simulink model "BaseEngineController_A02"  (Simulink 7.7)
settings: Solver=FixedStepDiscrete  StartTime=0.0  StopTime=10.0  RelTol=1e-3
PreLoadFcn:
  | try, eval([bdroot '_setup']); catch, eval('model_setup', ''); end
InitFcn:
  | try, eval([bdroot '_setup']); catch, eval('model_setup', ''); end
undefined symbols (not defined in the file or in its folder): ECU555, High, motohawk_build_version, motohawk_get_faults, motohawk_trunc_name, motohawk_vardec_config_group, motohawk_vardec_path, True
libraries: MotoHawk_lib x1145, AutoDoc_lib x786, motohawk_control_primitives_lib x114, motohawk_engine_lib x19, motohawk_annotations_lib x12, simulink x11, simulink_extras x1
(part 12 of 23)

== system BaseEngineController_A02/Foreground/Control/Controller/Run/Base Equiv Ratio ==
  Sensors : Inport
  Bus Creator : BusCreator  Inputs=2
  Bus Selector : BusSelector  OutputSignals=VirtualIndexes.IndTorqueNom17Idx,RawIndexes.RPM17Idx
  Bus Selector2 : BusSelector  OutputSignals=VirtualIndexes.IndPower05Idx,VirtualIndexes.NECCT09Idx
  Documentation_Calibrations_Probes_Block : AutoDoc_lib/Documentation_Calibrations_Probes_Block
  Documentation_Left_Border : AutoDoc_lib/Documentation_Left_Border  layout=Portrait
  Documentation_Right_Border : AutoDoc_lib/Documentation_Right_Border  layout=Portrait
  Documentation_Title_Block : AutoDoc_lib/Documentation_Title_Block
  Product1 : Product  RndMeth=Zero
  motohawk_interpolation_2d1 : MotoHawk_lib/Lookup Tables/motohawk_interpolation_2d  nam='WarmUpEqRatioMult'  table_data=ones(5,9)  show_more=on  data_type=Inherit from 'Table Data'  storage=NonAdaptive  read_level=1  write_level=1  ovr_cals=on  use_help=on  help='Multiplier on base eq. ratio as a function of NECCT and Ind Power.'  units='mult'  use_min_max=off  min_val=0  max_val=inf  use_precision=on  precision=''  gain=1  offset=0  exp=1  use_links=off  row_prelookup_block=''  col_prelookup_block=''  output_name=''  use_mototune_group=on  mototune_group=motohawk_vardec_path(gcb)
  motohawk_interpolation_2d3 : MotoHawk_lib/Lookup Tables/motohawk_interpolation_2d  nam='DesEquivRatio'  table_data=ones(17)  show_more=on  data_type=Inherit from 'Table Data'  storage=NonAdaptive  read_level=1  write_level=1  ovr_cals=on  use_help=on  help='Desired liquid equivalence ratio.'  units=''  use_min_max=on  min_val=0  max_val=inf  use_precision=on  precision=''  gain=1  offset=0  exp=1  use_links=off  row_prelookup_block=''  col_prelookup_block=''  output_name=''  use_mototune_group=on  mototune_group=motohawk_vardec_path(gcb)
  motohawk_override_abs : MotoHawk_lib/Calibration & Probing Blocks/motohawk_override_abs  SystemSampleTime=-1  parent_name='EquivRatio'  parent_val=0  show_more=on  name_source=Use Parameter  parent_read_level=1  parent_override_level=1  view_as=Number  enum_struct={'Disabled', 'Enabled'}  use_help_units=off  parent_help=''  parent_units=''  use_min_max=off  parent_min=-inf  parent_max=inf  use_precision=off  parent_precision=''  parent_gain=1  parent_offset=0  parent_exp=1  use_mototune_group=on  parent_group=motohawk_vardec_path(gcb)
  motohawk_probe : MotoHawk_lib/Calibration & Probing Blocks/motohawk_probe  nam='BaseEquivRatio'  show_more=on  name_source=Use Parameter  read_level=1  view_as=Number  enum_struct={'Disabled', 'Enabled'}  vector_style=Wide Row  use_help_units=on  help='Base equivalence ratio for selected fuel system.'  units=''  use_header_enum=off  row_header_enum={}  col_header_enum={}  use_precision=on  precision=''  gain=1  offset=0  exp=1  use_mototune_group=on  mototune_group=motohawk_vardec_path(gcb)
  EquivRatioInfo : Outport
  wires:
  Bus Selector/2 -> motohawk_interpolation_2d3/2  "<RPM17Idx>"
  Bus Selector/1 -> motohawk_interpolation_2d3/1  "<IndTorqueNom17Idx>"
  Sensors/1 -> Bus Selector/1, Bus Selector2/1
  Bus Selector2/1 -> motohawk_interpolation_2d1/1  "<IndPower05Idx>"
  Bus Selector2/2 -> motohawk_interpolation_2d1/2  "<NECCT09Idx>"
  Bus Creator/1 -> EquivRatioInfo/1
  Product1/1 -> motohawk_probe/1, Bus Creator/1  "DesEquivRatio"
  motohawk_override_abs/1 -> Product1/1
  motohawk_interpolation_2d3/1 -> motohawk_override_abs/1
  motohawk_interpolation_2d1/1 -> Bus Creator/2, Product1/2  "WarmUpMult"

== system BaseEngineController_A02/Foreground/Control/Controller/Run/EGR ==
  Sensors : Inport
  Bus Selector : BusSelector  OutputSignals=VirtualIndexes.IndTorqueNom09Idx,RawIndexes.RPM09Idx
  Bus Selector3 : BusSelector  OutputSignals=RawIndexes.ECT05Idx
  Product : Product  RndMeth=Zero
  motohawk_interpolation_1d : MotoHawk_lib/Lookup Tables/motohawk_interpolation_1d  nam='ECTEGRInterpolation'  table_data=[0,0,0,0,0]  show_more=on  data_type=Inherit from 'Table Data'  storage=NonAdaptive  read_level=1  write_level=1  ovr_cals=on  use_help=off  help=''  units=''  use_min_max=off  min_val=-inf  max_val=inf  use_precision=off  precision=''  gain=1  offset=0  exp=1  use_links=off  prelookup_block=''  output_name=''  use_mototune_group=on  mototune_group=motohawk_vardec_path(gcb)
  motohawk_interpolation_2d3 : MotoHawk_lib/Lookup Tables/motohawk_interpolation_2d  nam='EGR_Mult'  table_data=ones(9)  show_more=on  data_type=Inherit from 'Table Data'  storage=NonAdaptive  read_level=1  write_level=1  ovr_cals=on  use_help=on  help='Multiplier on ECT spark offset table.'  units='mult'  use_min_max=off  min_val=-inf  max_val=inf  use_precision=on  precision=''  gain=1  offset=0  exp=1  use_links=off  row_prelookup_block=''  col_prelookup_block=''  output_name=''  use_mototune_group=on  mototune_group='Engine Control | Run | Spark | Temp Comp'
  motohawk_probe : MotoHawk_lib/Calibration & Probing Blocks/motohawk_probe  nam='EGR_Percentage'  show_more=on  name_source=Use Parameter  read_level=1  view_as=Number  enum_struct={'Disabled', 'Enabled'}  vector_style=Wide Row  use_help_units=on  help='Total Spark Offset based on ECT, IAT2, engine speed and load'  units='degCA'  use_header_enum=off  row_header_enum={}  col_header_enum={}  use_precision=off  precision=''  gain=1  offset=0  exp=1  use_mototune_group=on  mototune_group='Engine Control | Run | Spark | Temp Comp'
  EGR% : Outport
  wires:
  Sensors/1 -> Bus Selector3/1, Bus Selector/1
  Bus Selector3/1 -> motohawk_interpolation_1d/1  "<ECT05Idx>"
  Bus Selector/1 -> motohawk_interpolation_2d3/1  "<IndTorqueNom09Idx>"
  Bus Selector/2 -> motohawk_interpolation_2d3/2  "<RPM09Idx>"
  motohawk_interpolation_2d3/1 -> Product/2  "RichEquivRatioTargetDelta"
  motohawk_interpolation_1d/1 -> Product/1
  Product/1 -> motohawk_probe/1, EGR%/1

== system BaseEngineController_A02/Foreground/Control/Controller/Run/Fuel System Manager ==
  Sensors : Inport
  O2_Info : Inport  Port=2
  Bus Selector1 : BusSelector  OutputSignals=DesiredLambda.WarmUpMult
  Documentation_Calibrations_Probes_Block : AutoDoc_lib/Documentation_Calibrations_Probes_Block
  Documentation_Left_Border : AutoDoc_lib/Documentation_Left_Border  layout=Portrait
  Documentation_Right_Border : AutoDoc_lib/Documentation_Right_Border  layout=Portrait
  Documentation_Title_Block : AutoDoc_lib/Documentation_Title_Block
  Fuel Calculation : Subsystem
      mask Display:
      | patch([0 0 1 1], 0.5*[0 0.075 0.075 0], [230/255 0/255 0/255])
      | patch([0 0 1 1], 0.5*[0.025 0.05 0.05 0.025], [150/255 0/255 0/255])
      | patch([0 0 1 1], 0.5*[0 0.025 0.025 0], [0/255 0/255 0/255])
      | 
      | disp('BASE FUEL');
  Injector Manager : Subsystem  TreatAsAtomicUnit=on
      mask Display:
      | patch([0 0 1 1], 0.5*[0 0.075 0.075 0], [230/255 0/255 0/255])
      | patch([0 0 1 1], 0.5*[0.025 0.05 0.05 0.025], [150/255 0/255 0/255])
      | patch([0 0 1 1], 0.5*[0 0.025 0.025 0], [0/255 0/255 0/255])
  O2 Fuel Adapt : Subsystem  TreatAsAtomicUnit=on
      mask Display:
      | patch([0 0 1 1], 0.5*[0 0.075 0.075 0], [230/255 0/255 0/255])
      | patch([0 0 1 1], 0.5*[0.025 0.05 0.05 0.025], [150/255 0/255 0/255])
      | patch([0 0 1 1], 0.5*[0 0.025 0.025 0], [0/255 0/255 0/255])
  DesiredFuel : Outport
  InjTiming : Outport  Port=2
  EOI : Outport  Port=3
  FuelMult : Outport  Port=4
  wires:
  O2_Info/1 -> Bus Selector1/1, O2 Fuel Adapt/1(O2 Info), Fuel Calculation/2(O2Info)
  Fuel Calculation/1(Fuel) -> Injector Manager/1(Fuel)
  Injector Manager/1(PrimaryInjTiming) -> InjTiming/1
  Injector Manager/2(MakeUpInjTiming) -> EOI/1
  Injector Manager/3(FPC) -> DesiredFuel/1
  O2 Fuel Adapt/1("Adapt Fuel Mult ") -> FuelMult/1
  Sensors/1 -> O2 Fuel Adapt/2(Sensors), Injector Manager/2(Sensors), Fuel Calculation/1(Sensors)
  Bus Selector1/1 -> O2 Fuel Adapt/3(WarmUp)  "<WarmUpMult>"

== system BaseEngineController_A02/Foreground/Control/Controller/Run/MaxGovernor ==
  MinGovInfo : Inport
  Sensors : Inport  Port=2
  Trigger : TriggerPort  TriggerType=function-call
  Bus Creator : BusCreator  Inputs=2
  Bus Selector1 : BusSelector  OutputSignals=BaseAirOfst
  Bus Selector2 : BusSelector  OutputSignals=RawProcessed.ETCRequest
  Bus Selector3 : BusSelector  OutputSignals=VirtualIndexes.RPMAccel17Idx
  Bus Selector4 : BusSelector  OutputSignals=Raw.RPMInst
  Bus Selector5 : BusSelector  OutputSignals=Raw.RPMInst,Raw.Digital.GearState
  Documentation_Calibrations_Probes_Block : AutoDoc_lib/Documentation_Calibrations_Probes_Block
  Documentation_Left_Border : AutoDoc_lib/Documentation_Left_Border  layout=Portrait
  Documentation_Right_Border : AutoDoc_lib/Documentation_Right_Border  layout=Portrait
  Documentation_Title_Block : AutoDoc_lib/Documentation_Title_Block
  I-Term Preload : Subsystem  TreatAsAtomicUnit=on
  MaxGovState : Subsystem
  Ramp RPM : Subsystem
      mask Display: disp('RAMP RPM\nINTO STEADY-STATE');
  Sum : Sum  Inputs=|++
  Terminator : Terminator
  Trigger Pre-load : Subsystem
  Unit Delay : UnitDelay  SampleTime=-1
  Universal PID (Tunable & Scheduled) : Subsystem  AncestorBlock=motohawk_control_primitives_lib/Universal PID (Tunable & Scheduled)  mask(Universal PID (Tunable)){nam='MaxGov', prefix='MaxGov', samp_time=-1, units='%', group=motohawk_vardec_path(gcb)}
      mask prompts: nam "Name"; samp_time "Sample Time"; group "MotoTune Group String"
      mask help:
      | This block wraps a 'Universal PID' block to provide gain scheduling and calibrations and probes for most parameters.
      |
      | If 'Sample Time' is -1, then extra code and RAM will be generated to dynamically calculate dt.
      mask init:
      | grp = [group ' | ' nam];
      mask Display:
      | patch([0 0 1 1], [0 0.075 0.075 0], [230/255 0/255 0/255])
      | patch([0 0 1 1], [0.025 0.05 0.05 0.025], [150/255 0/255 0/255])
      | patch([0 0 1 1], [0 0.025 0.025 0], [0/255 0/255 0/255])
      | 
      | disp([nam '\n(PID w/ Gain Schedules)']);
  motohawk_probe : MotoHawk_lib/Calibration & Probing Blocks/motohawk_probe  nam='TPSRequestLookupAirOfst'  show_more=on  name_source=Use Parameter  read_level=1  view_as=Number  enum_struct={'Disabled', 'Enabled'}  vector_style=Wide Row  use_help_units=on  help=''  units=''  use_header_enum=off  row_header_enum={}  col_header_enum={}  use_precision=on  precision=''  gain=1  offset=0  exp=1  use_mototune_group=on  mototune_group=motohawk_vardec_path(gcb)
  motohawk_probe2 : MotoHawk_lib/Calibration & Probing Blocks/motohawk_probe  nam='MaxGovOutput'  show_more=on  name_source=Use Parameter  read_level=1  view_as=Number  enum_struct={'Disabled', 'Enabled'}  vector_style=Wide Row  use_help_units=on  help=''  units=''  use_header_enum=off  row_header_enum={}  col_header_enum={}  use_precision=on  precision=''  gain=1  offset=0  exp=1  use_mototune_group=on  mototune_group=motohawk_vardec_path(gcb)
  MaxGovInfo : Outport
  wires:
  MinGovInfo/1 -> Bus Selector1/1
  Bus Creator/1 -> MaxGovInfo/1
  Bus Selector1/1 -> Sum/1  "<BaseAirOfst>"
  Bus Selector2/1 -> Sum/2  "<ETCRequest>"
  Sensors/1 -> Bus Selector2/1, Bus Selector5/1, Bus Selector3/1, Bus Selector4/1, MaxGovState/3(Sensors)
  Sum/1 -> I-Term Preload/1(TotalETCReq), MaxGovState/2(TotalETCReq), motohawk_probe/1
  Bus Selector3/1 -> I-Term Preload/2(RPMAccelIdx)  "<RPMAccel17Idx>"
  I-Term Preload/1(ITerm Preload) -> Universal PID (Tunable & Scheduled)/1(ITerm IC)
  MaxGovState/1(ETCMode) -> Bus Creator/1, Unit Delay/1  "MaxGovState"
  Trigger Pre-load/1(Trigger) -> MaxGovState/1(RPMEntryCriteria)
  Bus Selector4/1 -> Universal PID (Tunable & Scheduled)/5(Actual), Ramp RPM/3(RPM)  "<RPMInst>"
  Bus Selector5/1 -> Trigger Pre-load/1(RPM)  "<RPMInst>"
  Universal PID (Tunable & Scheduled)/1(MaxGovOut) -> Bus Creator/2, motohawk_probe2/1  "MaxGovPID"
  Universal PID (Tunable & Scheduled)/2(MaxGovITerm) -> Terminator/1
  Trigger Pre-load/2(BaseRPMSetpoint) -> Ramp RPM/2(TargetRPM), MaxGovState/4(TargetRPM)
  Unit Delay/1 -> Ramp RPM/1(OnIdle)
  MaxGovState/3(PIDHold) -> Universal PID (Tunable & Scheduled)/3(Hold PID)
  Ramp RPM/1(RPMSetPt) -> Universal PID (Tunable & Scheduled)/4(SetPoint)
  Bus Selector5/2 -> Trigger Pre-load/2(GearState)  "<GearState>"
  MaxGovState/2(PIDReset) -> Universal PID (Tunable & Scheduled)/2(Reset All)

== system BaseEngineController_A02/Foreground/Control/Controller/Run/MinGovernor ==
  Sensors : Inport
  Trigger : TriggerPort  TriggerType=function-call
  AiflowOffset : Subsystem  TreatAsAtomicUnit=on
  Base Airflow Table Adapt : Subsystem  TreatAsAtomicUnit=on
  Base RPM Set Pt : Subsystem  TreatAsAtomicUnit=on
  Bus Creator2 : BusCreator  Inputs=5
  Bus Selector : BusSelector  OutputSignals=Raw.RPM
  Bus Selector1 : BusSelector  OutputSignals=Raw.RPMInst
  Constant1 : Constant  Value=0  OutDataTypeStr=Inherit: Inherit via back propagation  SampleTime=-1
  Data Type Conversion1 : DataTypeConversion  SaturateOnIntegerOverflow=on  RndMeth=Zero
  Documentation_Calibrations_Probes_Block : AutoDoc_lib/Documentation_Calibrations_Probes_Block
  Documentation_Left_Border : AutoDoc_lib/Documentation_Left_Border  layout=Portrait
  Documentation_Right_Border : AutoDoc_lib/Documentation_Right_Border  layout=Portrait
  Documentation_Title_Block : AutoDoc_lib/Documentation_Title_Block
  Handoff Alpha : motohawk_control_primitives_lib/Handoff Alpha  SystemSampleTime=-1  samp_time=-1
  Hit  Crossing1 : HitCross  HitCrossingOffset=.5  HitCrossingDirection=falling
  Idle State Determination : Subsystem
      mask Display: disp('IDLE STATE\nDETERMINATION');
  Logical Operator : Logic  Operator=OR  AllPortsSameDT=off
  MotoringDetection : Subsystem
  OnIdle State : motohawk_control_primitives_lib/CompareTo  SystemSampleTime=-1  op===  val=0
  Product : Product  SaturateOnIntegerOverflow=on  InputSameDT=on  OutDataTypeStr=Inherit: Same as first input
  Product1 : Product  SaturateOnIntegerOverflow=on  InputSameDT=on  OutDataTypeStr=Inherit: Same as first input
  Ramp RPM : Subsystem
      mask Display: disp('RAMP RPM\nINTO IDLE STATE');
  Unit Delay1 : UnitDelay  SampleTime=-1
  Unit Delay2 : UnitDelay  SampleTime=-1
  Unit Delay3 : UnitDelay  SampleTime=-1
  Universal PD (Tunable & Scheduled) : Subsystem  AncestorBlock=motohawk_control_primitives_lib/Universal PD (Tunable & Scheduled)  mask(Universal PD (Tunable)){nam='SpkMinGov', prefix='SpkMinGov', samp_time=-1, units='degCA', group=motohawk_vardec_path(gcb)}
      mask prompts: nam "Name"; samp_time "Sample Time"; group "MotoTune Group String"
      mask help:
      | This block wraps a 'Universal PD' block to provide gain scheduling and calibrations and probes for most parameters.
      |
      | If 'Sample Time' is -1, then extra code and RAM will be generated to dynamically calculate dt.
      mask init:
      | grp = [group ' | ' nam];
      mask Display:
      | patch([0 0 1 1], [0 0.075 0.075 0], [230/255 0/255 0/255])
      | patch([0 0 1 1], [0.025 0.05 0.05 0.025], [150/255 0/255 0/255])
      | patch([0 0 1 1], [0 0.025 0.025 0], [0/255 0/255 0/255])
      | 
      | disp([nam '\n(PD w/ Gain Schedules)']);
  Universal PID (Tunable & Scheduled) : Subsystem  AncestorBlock=motohawk_control_primitives_lib/Universal PID (Tunable & Scheduled)  mask(Universal PID (Tunable)){nam='AirMinGov', prefix='AirMinGov', samp_time=-1, units='%', group=motohawk_vardec_path(gcb)}
      mask prompts: nam "Name"; samp_time "Sample Time"; group "MotoTune Group String"
      mask help:
      | This block wraps a 'Universal PID' block to provide gain scheduling and calibrations and probes for most parameters.
      |
      | If 'Sample Time' is -1, then extra code and RAM will be generated to dynamically calculate dt.
      mask init:
      | grp = [group ' | ' nam];
      mask Display:
      | patch([0 0 1 1], [0 0.075 0.075 0], [230/255 0/255 0/255])
      | patch([0 0 1 1], [0.025 0.05 0.05 0.025], [150/255 0/255 0/255])
      | patch([0 0 1 1], [0 0.025 0.025 0], [0/255 0/255 0/255])
      | 
      | disp([nam '\n(PID w/ Gain Schedules)']);
  motohawk_calibration3 : MotoHawk_lib/Calibration & Probing Blocks/motohawk_calibration  nam='IdleExitTimeConst'  val=0.005  storage=Calibration  show_more=on  name_source=Use Parameter  data_type=Inherit from 'Default Value'  read_level=1  write_level=1  ovr_cals=on  view_as=Number  enum_struct={'Disabled', 'Enabled'}  vector_style=Wide Row  use_help_units=on  help='Time constant for idle air and spark ramp out'  units='sec'  use_header_enum=off  row_header_enum={}  col_header_enum={}  use_min_max=off  min_val=0  max_val=100  use_precision=on  precision='2.3'  gain=1  offset=0  exp=1  use_mototune_group=on  mototune_group=motohawk_vardec_path(gcb)
  motohawk_probe2 : MotoHawk_lib/Calibration & Probing Blocks/motohawk_probe  nam='IdleHandOffMult'  show_more=on  name_source=Use Parameter  read_level=1  view_as=Number  enum_struct={'Disabled', 'Enabled'}  vector_style=Wide Row  use_help_units=off  help=''  units=''  use_header_enum=off  row_header_enum={}  col_header_enum={}  use_precision=off  precision=''  gain=1  offset=0  exp=1  use_mototune_group=on  mototune_group=motohawk_vardec_path(gcb)
  motohawk_probe3 : MotoHawk_lib/Calibration & Probing Blocks/motohawk_probe  nam='MinGovReset'  show_more=on  name_source=Use Parameter  read_level=1  view_as=Number  enum_struct={'Disabled', 'Enabled'}  vector_style=Wide Row  use_help_units=on  help=''  units=''  use_header_enum=off  row_header_enum={}  col_header_enum={}  use_precision=on  precision=''  gain=1  offset=0  exp=1  use_mototune_group=on  mototune_group=motohawk_vardec_path(gcb)
  motohawk_probe4 : MotoHawk_lib/Calibration & Probing Blocks/motohawk_probe  nam='AirMinGovOutput'  show_more=on  name_source=Use Parameter  read_level=1  view_as=Number  enum_struct={'Disabled', 'Enabled'}  vector_style=Wide Row  use_help_units=on  help='Total output from min. gov. air PID controller.'  units='%'  use_header_enum=off  row_header_enum={}  col_header_enum={}  use_precision=on  precision=''  gain=1  offset=0  exp=1  use_mototune_group=on  mototune_group=motohawk_vardec_path(gcb)
  motohawk_probe5 : MotoHawk_lib/Calibration & Probing Blocks/motohawk_probe  nam='SpkMinGovOutput'  show_more=on  name_source=Use Parameter  read_level=1  view_as=Number  enum_struct={'Disabled', 'Enabled'}  vector_style=Wide Row  use_help_units=on  help=''  units=''  use_header_enum=off  row_header_enum={}  col_header_enum={}  use_precision=on  precision=''  gain=1  offset=0  exp=1  use_mototune_group=on  mototune_group=motohawk_vardec_path(gcb)
  MinGovInfo : Outport
  wires:
  Sensors/1 -> Bus Selector1/1, Bus Selector/1, Base RPM Set Pt/1(Sensors), Idle State Determination/1(Sensors), AiflowOffset/1(Sensors)
  Bus Selector/1 -> Universal PD (Tunable & Scheduled)/1(Actual), Universal PID (Tunable & Scheduled)/1(Actual), MotoringDetection/1(ActualRPM)  "<RPM>"
  Idle State Determination/1(OnIdle) -> Bus Creator2/2, Ramp RPM/1(OnIdle), Base Airflow Table Adapt/Enable, Base Airflow Table Adapt/1(Idle State), Handoff Alpha/1  "OnIdle"
  Ramp RPM/1(RPMSetPt) -> Bus Creator2/3, Universal PD (Tunable & Scheduled)/2(SetPoint), Universal PID (Tunable & Scheduled)/2(SetPoint), MotoringDetection/2(SetpointRPM)  "MinGovSetpoint"
  Base RPM Set Pt/1(RPMSetPt) -> Idle State Determination/3(TargetRPM), Ramp RPM/2(TargetRPM)
  AiflowOffset/1(BaseAirOffset) -> Bus Creator2/1  "BaseAirOfst"
  Bus Selector1/1 -> Ramp RPM/3(RPM)  "<RPMInst>"
  MotoringDetection/1(MotoringFlag) -> Unit Delay2/1
  Unit Delay2/1 -> Hit  Crossing1/1
  Hit  Crossing1/1 -> Data Type Conversion1/1
  Universal PID (Tunable & Scheduled)/2(MinGovITerm) -> Unit Delay1/1
  Handoff Alpha/1 -> motohawk_probe2/1, Product/1, Product1/1, OnIdle State/1
  motohawk_calibration3/1 -> Handoff Alpha/3
  Constant1/1 -> Handoff Alpha/2
  Data Type Conversion1/1 -> Logical Operator/2
  Logical Operator/1 -> Universal PID (Tunable & Scheduled)/3(ResetAll), motohawk_probe3/1
  OnIdle State/1 -> Universal PD (Tunable & Scheduled)/3(ResetAll), Logical Operator/1
  Universal PD (Tunable & Scheduled)/1(SpkOut) -> Product/2
  Product/1 -> Bus Creator2/4, motohawk_probe5/1  "IdleSpk"
  Universal PID (Tunable & Scheduled)/1(MinGovOut) -> Product1/2
  Product1/1 -> Bus Creator2/5, Unit Delay3/1, motohawk_probe4/1  "MinGovAirPID"
  Unit Delay1/1 -> Base Airflow Table Adapt/2(ITerm)
  Unit Delay3/1 -> Idle State Determination/2(MinGovOutput)
  Bus Creator2/1 -> MinGovInfo/1  "MinGovInfo"

== system BaseEngineController_A02/Foreground/Control/Controller/Run/O2 Control ==
  Reset : Inport
  Sensors : Inport  Port=2
  Desired_Equiv : Inport  Port=3
  Bus Creator : BusCreator
  Bus Creator1 : BusCreator  Inputs=2
  Bus Creator2 : BusCreator  Inputs=1
  Bus Selector1 : BusSelector  OutputSignals=DesEquivRatio
  Bus Selector2 : BusSelector  OutputSignals=RawProcessed.O2Delagged.PreO2Delag
  Bus Selector4 : BusSelector  OutputSignals=TorqueCalcs.SSEng,VirtualIndexes.ECTKeyUp09Idx,RawProcessed.TimeSinceRun
  Documentation_Calibrations_Probes_Block : AutoDoc_lib/Documentation_Calibrations_Probes_Block
  Documentation_Left_Border : AutoDoc_lib/Documentation_Left_Border  layout=Portrait
  Documentation_Right_Border : AutoDoc_lib/Documentation_Right_Border  layout=Portrait
  Documentation_Title_Block : AutoDoc_lib/Documentation_Title_Block
  Logical Operator : Logic  Operator=NOT  AllPortsSameDT=off  OutDataTypeStr=boolean
  Logical Operator1 : Logic  Operator=NOT  AllPortsSameDT=off  OutDataTypeStr=boolean
  O2 Control State Determination : Subsystem
  O2 PID Calibrations : Subsystem
  O2 PID Controller : Subsystem  AncestorBlock=motohawk_engine_lib/O2A  TreatAsAtomicUnit=on  mask(Closed-Loop O2 Control){display_group='Engine Control | Run | Fuel | O2 Control', cal_group=motohawk_vardec_path(gcb)}
      mask prompts: display_group "MotoTune Display Group"; cal_group "MotoTune Calibration Group"
      mask Display:
      | patch([0 0 1 1], 0.5*[0 0.075 0.075 0], [230/255 0/255 0/255])
      | patch([0 0 1 1], 0.5*[0.025 0.05 0.05 0.025], [150/255 0/255 0/255])
      | patch([0 0 1 1], 0.5*[0 0.025 0.025 0], [0/255 0/255 0/255])
      | 
      | disp('Liquid O2 Control');
  motohawk_data_def : MotoHawk_lib/Data Storage Blocks/motohawk_data_def  nam='DisableO2FaultAction'  container_nam=''  data=0  typ=boolean  struct_nam='MyStruct'  storage=Volatile  outputRef=off  use_vardec=off  window=Calibration  read_level=1  write_level=1  ovr_cals=on  view_as=Number  enum_struct={'Disabled', 'Enabled'}  vector_style=Wide Row  use_help_units=on  help=''  units=''  use_header_enum=off  row_header_enum=''  col_header_enum=''  use_min_max=off  min_val=-inf  max_val=inf  use_precision=off  precision=''  gain=1  offset=0  exp=1  use_mototune_group=off  mototune_group=motohawk_vardec_path(gcb)  eval_name=DisableO2FaultAction  data_stores=motohawk_sfun_data_def_data_stores  struct_instances=motohawk_sfun_data_def_struct_instances  vardecs=motohawk_sfun_data_def_vardecs
  motohawk_data_write : MotoHawk_lib/Data Storage Blocks/motohawk_data_write  source=Lookup By Name  structure=Scalar  operation=Write scalar into location by index  sz_vec=-1  sz_row=3  sz_col=2  typ=boolean  nam='DisableO2FaultAction'  checkNull=on  write_read_only=off  struct_inst_name='Struct1'  struct_name='MyStruct'
  motohawk_fault_action : MotoHawk_lib/Fault Management Blocks/motohawk_fault_action  nam='DisableO2Ctrl'  output_status=on  eval_nam=DisableO2Ctrl
  motohawk_prelookup13 : MotoHawk_lib/Lookup Tables/motohawk_prelookup  nam='O2ITerm'  breakpoint_data=[-0.4 -0.31 -0.22 -0.13 -0.04 0.04 0.13 0.22 0.31]  show_more=on  data_type=Inherit from 'Table Data'  storage=Constant  read_level=1  write_level=1  ovr_cals=on  use_help=on  help='Index for O2 ITerm.'  units='mult'  use_min_max=off  min_val=-inf  max_val=inf  use_precision=off  precision=''  gain=1  offset=0  exp=1  use_links=on  input_name=''  use_mototune_group=on  mototune_group=motohawk_vardec_path(gcb)
  motohawk_probe4 : MotoHawk_lib/Calibration & Probing Blocks/motohawk_probe  nam='O2FuelMult'  show_more=on  name_source=Use Parameter  read_level=1  view_as=Number  enum_struct={'Disabled', 'Enabled'}  vector_style=Wide Row  use_help_units=on  help='Closed-loop O2 control fuel multiplier.'  units='mult'  use_header_enum=off  row_header_enum={}  col_header_enum={}  use_precision=off  precision=''  gain=1  offset=0  exp=1  use_mototune_group=on  mototune_group=motohawk_vardec_path(gcb)
  O2_Info : Outport
  wires:
  Logical Operator1/1 -> O2 PID Controller/5(Reset)
  Logical Operator/1 -> O2 PID Controller/1(Hold PID)
  Reset/1 -> Logical Operator1/1
  Bus Creator1/1 -> Bus Creator/4  "O2Error"
  Desired_Equiv/1 -> Bus Creator/1, Bus Selector1/1  "DesiredLambda"
  Sensors/1 -> Bus Selector2/1, O2 PID Calibrations/1(Sensors), Bus Selector4/1
  O2 Control State Determination/1(O2Control) -> Bus Creator2/1, Logical Operator/1  "BankAEnable"
  motohawk_prelookup13/1 -> Bus Creator1/2  "ITermIdx"
  Bus Creator2/1 -> Bus Creator/2  "O2ControlEnable"
  O2 PID Calibrations/1(Calibrations) -> O2 PID Controller/4(Calibrations)
  Bus Selector1/1 -> O2 Control State Determination/5(DesEquivRatio), O2 PID Controller/3(DesEquivRatio)  "<DesEquivRatio>"
  Bus Selector2/1 -> O2 PID Controller/2(O2Sensor)  "<PreO2Delag>"
  motohawk_fault_action/1 -> O2 Control State Determination/1(O2 Sensor Fault), motohawk_data_write/1
  Bus Selector4/1 -> O2 Control State Determination/2(SSEngOpr)  "<SSEng>"
  Bus Selector4/2 -> O2 Control State Determination/3(ECT_KeyUp_Idx)  "<ECTKeyUp09Idx>"
  Bus Selector4/3 -> O2 Control State Determination/4(TimeSinceRun)  "<TimeSinceRun>"
  Bus Creator/1 -> O2_Info/1  "O2Info"
  O2 PID Controller/1(FuelMult) -> Bus Creator/3, motohawk_probe4/1  "O2FuelMult"
  O2 PID Controller/2(O2 Error) -> motohawk_prelookup13/1, Bus Creator1/1  "O2ITerm"

== system BaseEngineController_A02/Foreground/Control/Controller/Run/O2 Heater ==
  Sensors : Inport
  Bus Selector : BusSelector  OutputSignals=TorqueCalcs.NECCT
  Chart : Stateflow chart  TreatAsAtomicUnit=on
  motohawk_calibration : MotoHawk_lib/Calibration & Probing Blocks/motohawk_calibration  nam='Heater_Off_Temp'  val=70  storage=Calibration  show_more=off  name_source=Use Parameter  data_type=Inherit from 'Default Value'  read_level=1  write_level=1  ovr_cals=on  view_as=Number  enum_struct={'Disabled', 'Enabled'}  vector_style=Wide Row  use_help_units=off  help=''  units=''  use_header_enum=off  row_header_enum={}  col_header_enum={}  use_min_max=off  min_val=-inf  max_val=inf  use_precision=off  precision=''  gain=1  offset=0  exp=1  use_mototune_group=off  mototune_group=motohawk_vardec_path(gcb)
  motohawk_probe : MotoHawk_lib/Calibration & Probing Blocks/motohawk_probe  nam='Oxygen_Heater_Prb'  show_more=off  name_source=Use Parameter  read_level=1  view_as=Number  enum_struct={'Disabled', 'Enabled'}  vector_style=Wide Row  use_help_units=off  help=''  units=''  use_header_enum=off  row_header_enum={}  col_header_enum={}  use_precision=off  precision=''  gain=1  offset=0  exp=1  use_mototune_group=off  mototune_group=motohawk_vardec_path(gcb)
  Heater ON : Outport
  wires:
  Sensors/1 -> Bus Selector/1
  Bus Selector/1 -> Chart/1(NEECT)  "<NECCT>"
  motohawk_calibration/1 -> Chart/2(CAL)
  Chart/1(HEATER) -> Heater ON/1, motohawk_probe/1

== system BaseEngineController_A02/Foreground/Control/Controller/Run/Spark Manager ==
  DesEquivRatio : Inport
  Sensors : Inport  Port=2
  RPM_Ctrl_Ofst : Inport  Port=3
  Trigger : TriggerPort  TriggerType=function-call
  Apply Offsets to Base Spark Advance1 : Sum  SaturateOnIntegerOverflow=on  InputSameDT=on
  Apply Offsets to Base Spark Advance3 : Sum  Inputs=+++++  SaturateOnIntegerOverflow=on  InputSameDT=on
  Bus Selector : BusSelector  OutputSignals=VirtualIndexes.IndTorqueNom09Idx,RawIndexes.RPM09Idx
  Bus Selector1 : BusSelector  OutputSignals=VirtualIndexes.IndTorqueNom17Idx,RawIndexes.RPM17Idx
  Bus Selector2 : BusSelector  OutputSignals=IdleSpk
  Bus Selector3 : BusSelector  OutputSignals=DesEquivRatio
  Bus Selector4 : BusSelector  OutputSignals=OnIdle
  Desired Eq Ratio Offset : Subsystem
  Documentation_Calibrations_Probes_Block : AutoDoc_lib/Documentation_Calibrations_Probes_Block
  Documentation_Left_Border : AutoDoc_lib/Documentation_Left_Border  layout=Portrait
  Documentation_Right_Border : AutoDoc_lib/Documentation_Right_Border  layout=Portrait
  Documentation_Title_Block : AutoDoc_lib/Documentation_Title_Block
  EGR Spark Offset : Subsystem
  Ethanol Spark Offset : Subsystem
  Spark Advance Rate Limiter : Subsystem
  SparkAdvanceLimiter : Subsystem
  Temp Comp Offset : Subsystem
  motohawk_interpolation_2d1 : MotoHawk_lib/Lookup Tables/motohawk_interpolation_2d  nam='BaseSparkEnergy'  table_data=ones(9)  show_more=on  data_type=Inherit from 'Table Data'  storage=NonAdaptive  read_level=1  write_level=1  ovr_cals=on  use_help=on  help='Spark energy - Run state'  units='mJ'  use_min_max=off  min_val=-inf  max_val=inf  use_precision=on  precision=''  gain=1  offset=0  exp=1  use_links=off  row_prelookup_block=''  col_prelookup_block=''  output_name=''  use_mototune_group=on  mototune_group=motohawk_vardec_path(gcb)
  motohawk_interpolation_2d2 : MotoHawk_lib/Lookup Tables/motohawk_interpolation_2d  nam='SparkAdv'  table_data=ones(17)*10  show_more=on  data_type=Inherit from 'Table Data'  storage=NonAdaptive  read_level=1  write_level=1  ovr_cals=on  use_help=on  help='Base spark advance map.'  units='degBTDC'  use_min_max=on  min_val=-inf  max_val=inf  use_precision=on  precision=''  gain=1  offset=0  exp=1  use_links=off  row_prelookup_block=''  col_prelookup_block=''  output_name=''  use_mototune_group=on  mototune_group=motohawk_vardec_path(gcb)
  motohawk_probe : MotoHawk_lib/Calibration & Probing Blocks/motohawk_probe  nam='Spark_Adv_Prb'  show_more=off  name_source=Use Parameter  read_level=1  view_as=Number  enum_struct={'Disabled', 'Enabled'}  vector_style=Wide Row  use_help_units=off  help=''  units=''  use_header_enum=off  row_header_enum={}  col_header_enum={}  use_precision=off  precision=''  gain=1  offset=0  exp=1  use_mototune_group=off  mototune_group=motohawk_vardec_path(gcb)
  SparkEnergy : Outport
  SpkAdv : Outport  Port=2
  wires:
  SparkAdvanceLimiter/1(SparkAdv) -> Spark Advance Rate Limiter/1(DesSparkAdv), motohawk_probe/1
  Sensors/1 -> Bus Selector/1, Bus Selector1/1, Temp Comp Offset/1(Sensors), Desired Eq Ratio Offset/1(Sensors), Ethanol Spark Offset/1(Sensors), SparkAdvanceLimiter/3(Sensors), EGR Spark Offset/1(Sensors)
  Bus Selector4/1 -> SparkAdvanceLimiter/2(OnIdle)  "<OnIdle>"
  Apply Offsets to Base Spark Advance1/1 -> SparkAdvanceLimiter/1(DesSparkAdv)
  Spark Advance Rate Limiter/1(SparkAdv) -> SpkAdv/1
  Bus Selector/1 -> motohawk_interpolation_2d1/1  "<IndTorqueNom09Idx>"
  Bus Selector/2 -> motohawk_interpolation_2d1/2  "<RPM09Idx>"
  Bus Selector1/1 -> motohawk_interpolation_2d2/1  "<IndTorqueNom17Idx>"
  Bus Selector1/2 -> motohawk_interpolation_2d2/2  "<RPM17Idx>"
  Temp Comp Offset/1(TempSpkOfst) -> Apply Offsets to Base Spark Advance3/2
  Desired Eq Ratio Offset/1(EqRatioSpkOfst) -> Apply Offsets to Base Spark Advance3/3
  Apply Offsets to Base Spark Advance3/1 -> Apply Offsets to Base Spark Advance1/1
  RPM_Ctrl_Ofst/1 -> Bus Selector2/1, Bus Selector4/1
  Bus Selector2/1 -> Apply Offsets to Base Spark Advance1/2  "<IdleSpk>"
  DesEquivRatio/1 -> Bus Selector3/1
  Bus Selector3/1 -> Desired Eq Ratio Offset/2(DesiredEqRatio)  "<DesEquivRatio>"
  motohawk_interpolation_2d1/1 -> SparkEnergy/1
  motohawk_interpolation_2d2/1 -> Apply Offsets to Base Spark Advance3/1  "RichEquivRatioTargetDelta"
  Ethanol Spark Offset/1(EthanolSpkOfst) -> Apply Offsets to Base Spark Advance3/4
  EGR Spark Offset/1(EGRSpkOfst) -> Apply Offsets to Base Spark Advance3/5

== system BaseEngineController_A02/Foreground/Control/Controller/Stall/Fuel Pump at Startup ==
  note: Turn On Fuel Pump
      | for 'StartupTime' seconds
  note: Turn on Fuel Pump at Startup
  Documentation_Calibrations_Probes_Block : AutoDoc_lib/Documentation_Calibrations_Probes_Block
  Documentation_Left_Border : AutoDoc_lib/Documentation_Left_Border  layout=Portrait
  Documentation_Right_Border : AutoDoc_lib/Documentation_Right_Border  layout=Portrait
  Documentation_Title_Block : AutoDoc_lib/Documentation_Title_Block
  Relational Operator : RelationalOperator  Operator=<  ZeroCross=off  InputSameDT=on
  Time Since Enabled : motohawk_control_primitives_lib/Time Since Enabled  SystemSampleTime=-1  samp_time=.005
  motohawk_calibration : MotoHawk_lib/Calibration & Probing Blocks/motohawk_calibration  nam='FuelPumpStartTime'  val=3  storage=Calibration  show_more=on  name_source=Use Parameter  data_type=Inherit from 'Default Value'  read_level=1  write_level=1  ovr_cals=on  view_as=Number  enum_struct={'Disabled', 'Enabled'}  vector_style=Wide Row  use_help_units=on  help='The length of time for the Fuel Pump relay to be enabled at startup'  units='sec'  use_header_enum=off  row_header_enum={}  col_header_enum={}  use_min_max=on  min_val=0  max_val=100  use_precision=off  precision=''  gain=1  offset=0  exp=1  use_mototune_group=on  mototune_group=motohawk_vardec_path(gcb)
  motohawk_probe : MotoHawk_lib/Calibration & Probing Blocks/motohawk_probe  nam='FuelPumpTSE'  show_more=on  name_source=Use Parameter  read_level=1  view_as=Number  enum_struct={'Disabled', 'Enabled'}  vector_style=Wide Row  use_help_units=on  help=''  units=''  use_header_enum=off  row_header_enum={}  col_header_enum={}  use_precision=on  precision=''  gain=1  offset=0  exp=1  use_mototune_group=on  mototune_group=motohawk_vardec_path(gcb)
  FUELP [bool] : Outport
  wires:
  Time Since Enabled/1 -> Relational Operator/1, motohawk_probe/1
  motohawk_calibration/1 -> Relational Operator/2
  Relational Operator/1 -> FUELP [bool]/1

== system BaseEngineController_A02/Foreground/Control/Controller/Stall/Oil Pump at Startup ==
  note: Turn On Fuel Pump
      | for 'StartupTime' seconds
  note: Turn on Fuel Pump at Startup
  Documentation_Calibrations_Probes_Block : AutoDoc_lib/Documentation_Calibrations_Probes_Block
  Documentation_Left_Border : AutoDoc_lib/Documentation_Left_Border  layout=Portrait
  Documentation_Right_Border : AutoDoc_lib/Documentation_Right_Border  layout=Portrait
  Documentation_Title_Block : AutoDoc_lib/Documentation_Title_Block
  Relational Operator : RelationalOperator  Operator=<  ZeroCross=off  InputSameDT=on
  Time Since Enabled : motohawk_control_primitives_lib/Time Since Enabled  SystemSampleTime=-1  samp_time=.005
  motohawk_calibration : MotoHawk_lib/Calibration & Probing Blocks/motohawk_calibration  nam='OilPumpStartTime'  val=3  storage=Calibration  show_more=on  name_source=Use Parameter  data_type=Inherit from 'Default Value'  read_level=1  write_level=1  ovr_cals=on  view_as=Number  enum_struct={'Disabled', 'Enabled'}  vector_style=Wide Row  use_help_units=on  help='The length of time for the Fuel Pump relay to be enabled at startup'  units='sec'  use_header_enum=off  row_header_enum={}  col_header_enum={}  use_min_max=on  min_val=0  max_val=100  use_precision=off  precision=''  gain=1  offset=0  exp=1  use_mototune_group=on  mototune_group=motohawk_vardec_path(gcb)
  motohawk_probe : MotoHawk_lib/Calibration & Probing Blocks/motohawk_probe  nam='OilPumpTSE'  show_more=on  name_source=Use Parameter  read_level=1  view_as=Number  enum_struct={'Disabled', 'Enabled'}  vector_style=Wide Row  use_help_units=on  help=''  units=''  use_header_enum=off  row_header_enum={}  col_header_enum={}  use_precision=on  precision=''  gain=1  offset=0  exp=1  use_mototune_group=on  mototune_group=motohawk_vardec_path(gcb)
  OILP [bool] : Outport
  wires:
  Time Since Enabled/1 -> Relational Operator/1, motohawk_probe/1
  motohawk_calibration/1 -> Relational Operator/2
  Relational Operator/1 -> OILP [bool]/1

== system BaseEngineController_A02/Foreground/Control/Diagnostics/Freeze Frames/Clear FFIDs and FltOrder ==
  Trigger : TriggerPort
  Constant : Constant  Value=uint16(0)
  Constant1 : Constant  Value=zeros(6,1)  OutDataTypeStr=uint8
  Constant2 : Constant  Value=zeros(length(motohawk_get_faults(bdroot)),1)  OutDataTypeStr=uint16
  Documentation_Calibrations_Probes_Block : AutoDoc_lib/Documentation_Calibrations_Probes_Block
  Documentation_Left_Border : AutoDoc_lib/Documentation_Left_Border  layout=Portrait
  Documentation_Right_Border : AutoDoc_lib/Documentation_Right_Border  layout=Portrait
  Documentation_Title_Block : AutoDoc_lib/Documentation_Title_Block
  motohawk_data_write1 : MotoHawk_lib/Data Storage Blocks/motohawk_data_write  source=Lookup By Name  structure=Vector  operation=Write entire data structure at once  sz_vec=6  sz_row=10  sz_col=1  typ=uint8  nam='FFIDs'  checkNull=on  write_read_only=off  struct_inst_name='Struct1'  struct_name='MyStruct'
  motohawk_data_write2 : MotoHawk_lib/Data Storage Blocks/motohawk_data_write  source=Lookup By Name  structure=Vector  operation=Write entire data structure at once  sz_vec=length(motohawk_get_faults(bdroot))  sz_row=10  sz_col=1  typ=uint16  nam='FltOrder'  checkNull=on  write_read_only=off  struct_inst_name='Struct1'  struct_name='MyStruct'
  motohawk_data_write3 : MotoHawk_lib/Data Storage Blocks/motohawk_data_write  source=Lookup By Name  structure=Scalar  operation=Write entire data structure at once  sz_vec=10  sz_row=10  sz_col=1  typ=uint16  nam='FltOrderMax'  checkNull=on  write_read_only=off  struct_inst_name='Struct1'  struct_name='MyStruct'
  wires:
  Constant/1 -> motohawk_data_write3/1
  Constant1/1 -> motohawk_data_write1/1
  Constant2/1 -> motohawk_data_write2/1

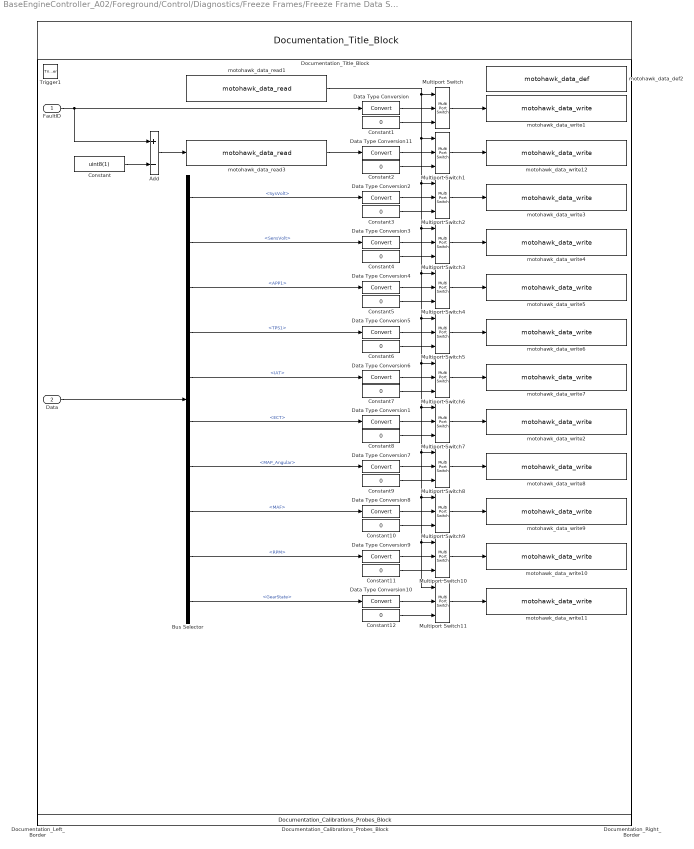
== system BaseEngineController_A02/Foreground/Control/Diagnostics/Freeze Frames/Freeze Frame Data Storage ==
  FaultID : Inport
  Data : Inport  Port=2
  Trigger1 : TriggerPort
  Add : Sum  Inputs=+-  OutDataTypeStr=Inherit: Same as first input
  Bus Selector : BusSelector  OutputSignals=SynthSensors.Raw.LinearSensors.SysVolt,SynthSensors.Raw.LinearSensors.SensVolt,SynthSensors.Raw.PotSensors.APP1,SynthSensors.Raw.PotSensors.TPS1,SynthSensors.Raw.NonLinearSensors.IAT,SynthSensors.Raw.NonLinearSensors.ECT,SynthSensors.Raw.LinearSensors.MAP_Angular,SynthSensors.Raw.Digital.MAF,SynthSensors.Raw.RPM,SynthSensors.Raw.Digital.GearState
  Constant : Constant  Value=uint8(1)
  Constant{1..12} (12 alike) : Constant  Value=0  OutDataTypeStr=Inherit: Inherit via back propagation
  Data Type Conversion{,1..11} (12 alike) : DataTypeConversion
  Documentation_Calibrations_Probes_Block : AutoDoc_lib/Documentation_Calibrations_Probes_Block
  Documentation_Left_Border : AutoDoc_lib/Documentation_Left_Border  layout=Portrait
  Documentation_Right_Border : AutoDoc_lib/Documentation_Right_Border  layout=Portrait
  Documentation_Title_Block : AutoDoc_lib/Documentation_Title_Block
  Multiport Switch{,1..11} (12 alike) : MultiPortSwitch  DataPortOrder=Zero-based contiguous  Inputs=2  zeroidx=on  InputSameDT=on
  motohawk_data_def2 : MotoHawk_lib/Data Storage Blocks/motohawk_data_def  nam=['FF' num2str(FFnum)]  container_nam='FreezeFramesContainer'  data=struct('Name', int32(0), 'Code', int32(0), 'Var1', int32(0), 'Var2', int32(0), 'Var3', int32(0), 'Var4', int32(0), 'Var5', int32(0), 'Var6', int32(0), 'Var7', int32(0), 'Var8', int32(0), 'Var9', int32(0), 'Var10', int32(0))  typ=struct  struct_nam='FreezeFrames'  storage=NonVolatile  outputRef=off  use_vardec=off  window=Display  read_level=1  write_level=(Read Only)  ovr_cals=on  view_as=Number  enum_struct={'Disabled', 'Enabled'}  vector_style=Wide Row  use_help_units=off  help=''  units=''  use_header_enum=off  row_header_enum={}  col_header_enum={}  use_min_max=off  min_val=-inf  max_val=inf  use_precision=off  precision=''  gain=1  offset=0  exp=1  use_mototune_group=off  mototune_group=''  eval_name=FF1  data_stores=motohawk_sfun_data_def_data_stores  struct_instances=motohawk_sfun_data_def_struct_instances  vardecs=motohawk_sfun_data_def_vardecs
  motohawk_data_read1 : MotoHawk_lib/Data Storage Blocks/motohawk_data_read  source=Lookup By Name  structure=Scalar  operation=Read scalar from location by index  sz_vec=3  sz_row=2  sz_col=3  typ=boolean  nam='ClearFreezeFrames'  checkNull=on  struct_inst_name='Struct1'  struct_nam='MyStruct'
  motohawk_data_read3 : MotoHawk_lib/Data Storage Blocks/motohawk_data_read  source=Lookup By Name  structure=Vector  operation=Read scalar from location by index  sz_vec=3  sz_row=2  sz_col=3  typ=uint16  nam='FltCodes'  checkNull=on  struct_inst_name='Struct1'  struct_nam='MyStruct'
  motohawk_data_write1 : MotoHawk_lib/Data Storage Blocks/motohawk_data_write  source=Lookup By Name in Structure  structure=Scalar  operation=Write entire data structure at once  sz_vec=len  sz_row=10  sz_col=1  typ=int32  nam='Name'  checkNull=on  write_read_only=off  struct_inst_name=['FF' num2str(FFnum)]  struct_name='FreezeFrames'
  motohawk_data_write10 : MotoHawk_lib/Data Storage Blocks/motohawk_data_write  source=Lookup By Name in Structure  structure=Scalar  operation=Write entire data structure at once  sz_vec=len  sz_row=10  sz_col=1  typ=int32  nam='Var9'  checkNull=on  write_read_only=off  struct_inst_name=['FF' num2str(FFnum)]  struct_name='FreezeFrames'
  motohawk_data_write11 : MotoHawk_lib/Data Storage Blocks/motohawk_data_write  source=Lookup By Name in Structure  structure=Scalar  operation=Write entire data structure at once  sz_vec=len  sz_row=10  sz_col=1  typ=int32  nam='Var10'  checkNull=on  write_read_only=off  struct_inst_name=['FF' num2str(FFnum)]  struct_name='FreezeFrames'
  motohawk_data_write12 : MotoHawk_lib/Data Storage Blocks/motohawk_data_write  source=Lookup By Name in Structure  structure=Scalar  operation=Write entire data structure at once  sz_vec=len  sz_row=10  sz_col=1  typ=int32  nam='Code'  checkNull=on  write_read_only=off  struct_inst_name=['FF' num2str(FFnum)]  struct_name='FreezeFrames'
  motohawk_data_write2 : MotoHawk_lib/Data Storage Blocks/motohawk_data_write  source=Lookup By Name in Structure  structure=Scalar  operation=Write entire data structure at once  sz_vec=len  sz_row=10  sz_col=1  typ=int32  nam='Var6'  checkNull=on  write_read_only=off  struct_inst_name=['FF' num2str(FFnum)]  struct_name='FreezeFrames'
  motohawk_data_write3 : MotoHawk_lib/Data Storage Blocks/motohawk_data_write  source=Lookup By Name in Structure  structure=Scalar  operation=Write entire data structure at once  sz_vec=len  sz_row=10  sz_col=1  typ=int32  nam='Var1'  checkNull=on  write_read_only=off  struct_inst_name=['FF' num2str(FFnum)]  struct_name='FreezeFrames'
  motohawk_data_write4 : MotoHawk_lib/Data Storage Blocks/motohawk_data_write  source=Lookup By Name in Structure  structure=Scalar  operation=Write entire data structure at once  sz_vec=len  sz_row=10  sz_col=1  typ=int32  nam='Var2'  checkNull=on  write_read_only=off  struct_inst_name=['FF' num2str(FFnum)]  struct_name='FreezeFrames'
  motohawk_data_write5 : MotoHawk_lib/Data Storage Blocks/motohawk_data_write  source=Lookup By Name in Structure  structure=Scalar  operation=Write entire data structure at once  sz_vec=len  sz_row=10  sz_col=1  typ=int32  nam='Var3'  checkNull=on  write_read_only=off  struct_inst_name=['FF' num2str(FFnum)]  struct_name='FreezeFrames'
  motohawk_data_write6 : MotoHawk_lib/Data Storage Blocks/motohawk_data_write  source=Lookup By Name in Structure  structure=Scalar  operation=Write entire data structure at once  sz_vec=len  sz_row=10  sz_col=1  typ=int32  nam='Var4'  checkNull=on  write_read_only=off  struct_inst_name=['FF' num2str(FFnum)]  struct_name='FreezeFrames'
  motohawk_data_write7 : MotoHawk_lib/Data Storage Blocks/motohawk_data_write  source=Lookup By Name in Structure  structure=Scalar  operation=Write entire data structure at once  sz_vec=len  sz_row=10  sz_col=1  typ=int32  nam='Var5'  checkNull=on  write_read_only=off  struct_inst_name=['FF' num2str(FFnum)]  struct_name='FreezeFrames'
  motohawk_data_write8 : MotoHawk_lib/Data Storage Blocks/motohawk_data_write  source=Lookup By Name in Structure  structure=Scalar  operation=Write entire data structure at once  sz_vec=len  sz_row=10  sz_col=1  typ=int32  nam='Var7'  checkNull=on  write_read_only=off  struct_inst_name=['FF' num2str(FFnum)]  struct_name='FreezeFrames'
  motohawk_data_write9 : MotoHawk_lib/Data Storage Blocks/motohawk_data_write  source=Lookup By Name in Structure  structure=Scalar  operation=Write entire data structure at once  sz_vec=len  sz_row=10  sz_col=1  typ=int32  nam='Var8'  checkNull=on  write_read_only=off  struct_inst_name=['FF' num2str(FFnum)]  struct_name='FreezeFrames'
  wires:
  FaultID/1 -> Data Type Conversion/1, Add/1
  Bus Selector/1 -> Data Type Conversion2/1  "<SysVolt>"
  Bus Selector/2 -> Data Type Conversion3/1  "<SensVolt>"
  Bus Selector/3 -> Data Type Conversion4/1  "<APP1>"
  Bus Selector/4 -> Data Type Conversion5/1  "<TPS1>"
  Bus Selector/5 -> Data Type Conversion6/1  "<IAT>"
  Data/1 -> Bus Selector/1
  Bus Selector/6 -> Data Type Conversion1/1  "<ECT>"
  Bus Selector/7 -> Data Type Conversion7/1  "<MAP_Angular>"
  Bus Selector/8 -> Data Type Conversion8/1  "<MAF>"
  Bus Selector/9 -> Data Type Conversion9/1  "<RPM>"
  Bus Selector/10 -> Data Type Conversion10/1  "<GearState>"
  motohawk_data_read3/1 -> Data Type Conversion11/1
  Constant/1 -> Add/2
  Add/1 -> motohawk_data_read3/1
  Data Type Conversion/1 -> Multiport Switch/2
  Constant1/1 -> Multiport Switch/3
  motohawk_data_read1/1 -> Multiport Switch/1, Multiport Switch1/1, Multiport Switch2/1, Multiport Switch3/1, Multiport Switch4/1, Multiport Switch5/1, Multiport Switch6/1, Multiport Switch7/1, Multiport Switch8/1, Multiport Switch9/1, Multiport Switch11/1, Multiport Switch10/1
  Multiport Switch/1 -> motohawk_data_write1/1
  Multiport Switch1/1 -> motohawk_data_write12/1
  Constant2/1 -> Multiport Switch1/3
  Data Type Conversion11/1 -> Multiport Switch1/2
  Constant3/1 -> Multiport Switch2/3
  Constant4/1 -> Multiport Switch3/3
  Constant5/1 -> Multiport Switch4/3
  Constant6/1 -> Multiport Switch5/3
  Constant7/1 -> Multiport Switch6/3
  Constant8/1 -> Multiport Switch7/3
  Constant9/1 -> Multiport Switch8/3
  Constant10/1 -> Multiport Switch9/3
  Constant11/1 -> Multiport Switch10/3
  Constant12/1 -> Multiport Switch11/3
  Multiport Switch2/1 -> motohawk_data_write3/1
  Multiport Switch3/1 -> motohawk_data_write4/1
  Multiport Switch4/1 -> motohawk_data_write5/1
  Multiport Switch5/1 -> motohawk_data_write6/1
  Multiport Switch6/1 -> motohawk_data_write7/1
  Multiport Switch7/1 -> motohawk_data_write2/1
  Multiport Switch8/1 -> motohawk_data_write8/1
  Multiport Switch9/1 -> motohawk_data_write9/1
  Multiport Switch10/1 -> motohawk_data_write10/1
  Multiport Switch11/1 -> motohawk_data_write11/1
  Data Type Conversion2/1 -> Multiport Switch2/2
  Data Type Conversion3/1 -> Multiport Switch3/2
  Data Type Conversion4/1 -> Multiport Switch4/2
  Data Type Conversion5/1 -> Multiport Switch5/2
  Data Type Conversion6/1 -> Multiport Switch6/2
  Data Type Conversion1/1 -> Multiport Switch7/2
  Data Type Conversion7/1 -> Multiport Switch8/2
  Data Type Conversion8/1 -> Multiport Switch9/2
  Data Type Conversion9/1 -> Multiport Switch10/2
  Data Type Conversion10/1 -> Multiport Switch11/2
